simulink model "sim_discrete_planar_2dof"  (R2022b)
settings: Solver=VariableStepAuto  StartTime=0.0  StopTime=T_sim  FixedStep=param_global.Ta  RelTol=1e-3
InitFcn:
  | parameters_2dof;
undefined symbols (not defined in the file): coord_xaxis_len, coord_yaxis_len, coord_zaxis_len, fx, fy, fz, init_bus_param, param_global, param_path_set_points, param_robot, param_vis, path_coord_xaxis_len, path_coord_yaxis_len, path_coord_zaxis_len, path_param, q_0, q_0_p, tau_x, tau_y, tau_z, X, Y, Z
libraries: sm_lib x1241, nesl_utility x796
(part 1 of 34)
settings Model: Creator=emu  LastModifiedBy=emu
settings OptimizationCC: ReuseModelBlockBuffer=on
settings DebuggingCC: ReadBeforeWriteMsg=DisableAll  WriteAfterWriteMsg=DisableAll  WriteAfterReadMsg=DisableAll  MultiTaskDSMMsg=none
settings ModelReferenceCC: UpdateModelReferenceTargets=IfOutOfDate
settings SFSimCC: SFSimEcho=off

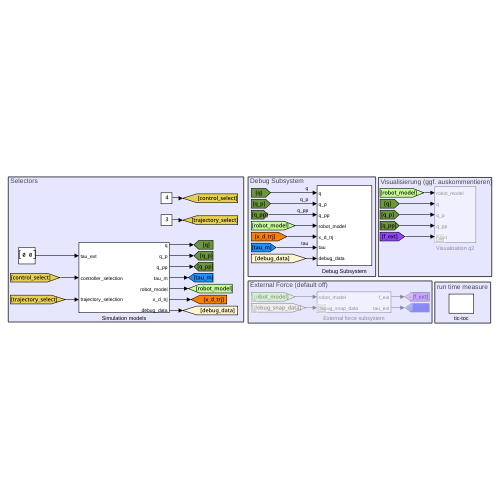
[thumbnail: Simulink's stored preview of the top-level diagram]
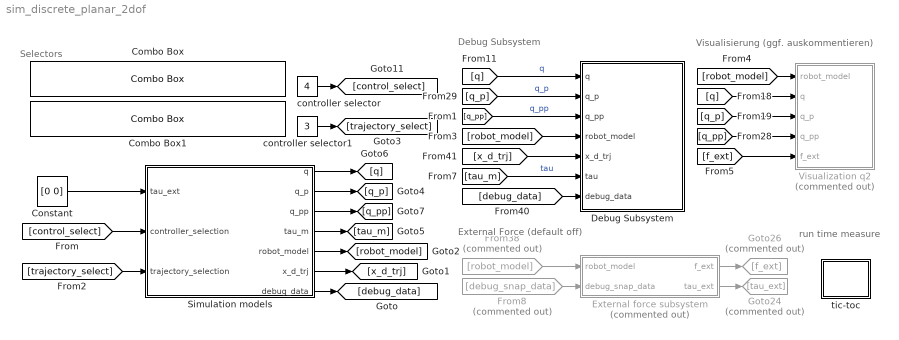
== system sim_discrete_planar_2dof ==
  note: run time measure
  note: Selectors
  note: Visualisierung (ggf. auskommentieren)
  note: Debug Subsystem
  note: External Force (default off)
  Combo Box : ComboBox  SelectedLabel=MPC 3 (qrqp, N=5, 50ms, v3)
      settings: Values={{'Cartesian CT Control', 'MPC 1 (ipopt, N=5, 20ms, v2)', 'MPC 2 (qrqp, N=5, 20ms, v2)', 'MPC 3 (qrqp, N=5, 50ms, v3)', 'MPC 4 (qrqp, N=5, 1ms, v1)', 'MPC 5 (qrqp, N=5, 10ms, v4'}, [1 2 3 4 5 6]}
  Combo Box1 : ComboBox  SelectedLabel=3: 5th order polynomial
      settings: Values={{'1: stabilize equilibrium', '2: 5th order differential filter', '3: 5th order polynomial', '4: smooth sinus'}, [1 2 3 4]}
  Constant : Constant  Value=[0 0]
  Debug Subsystem : Subsystem
  External force subsystem : Subsystem  Commented=on
  From38 : From  Commented=on  GotoTag=robot_model
  From8 : From  Commented=on  GotoTag=debug_snap_data
  Goto24 : Goto  Commented=on  GotoTag=tau_ext
  Goto26 : Goto  Commented=on  GotoTag=f_ext
  Simulation models : Subsystem
  Visualization q2 : Subsystem  Commented=on
  controller selector : Constant  Value=4  OutDataTypeStr=uint32  SampleTime=-1
  controller selector1 : Constant  Value=3  OutDataTypeStr=uint32  SampleTime=-1
  tic-toc : Subsystem
  wires:
  controller selector/1 -> [control_select]
  [tau_m] -> Debug Subsystem/6(tau)  "tau"
  [debug_data] -> Debug Subsystem/7(debug_data)
  External force subsystem/1(f_ext) -> Goto26/1
  [q_p] -> Debug Subsystem/2(q_p)  "q_p"
  [robot_model] -> Debug Subsystem/4(robot_model)
  [robot_model] -> Visualization q2/1(robot_model)
  From8/1 -> External force subsystem/2(debug_snap_data)
  [q_pp] -> Visualization q2/4(q_pp)
  [f_ext] -> Visualization q2/5(f_ext)
  [x_d_trj] -> Debug Subsystem/5(x_d_trj)
  From38/1 -> External force subsystem/1(robot_model)
  [q_p] -> Visualization q2/3(q_p)
  External force subsystem/2(tau_ext) -> Goto24/1
  [q] -> Visualization q2/2(q)
  [q] -> Debug Subsystem/1(q)  "q"
  [q_pp] -> Debug Subsystem/3(q_pp)  "q_pp"
  Simulation models/7(debug_data) -> [debug_data]
  Simulation models/6(x_d_trj) -> [x_d_trj]
  Simulation models/5(robot_model) -> [robot_model]
  Simulation models/4(tau_m) -> [tau_m]
  Simulation models/3(q_pp) -> [q_pp]
  Simulation models/2(q_p) -> [q_p]
  Simulation models/1(q) -> [q]
  [control_select] -> Simulation models/2(controller_selection)
  Constant/1 -> Simulation models/1(tau_ext)
  controller selector1/1 -> [trajectory_select]
  [trajectory_select] -> Simulation models/3(trajectory_selection)

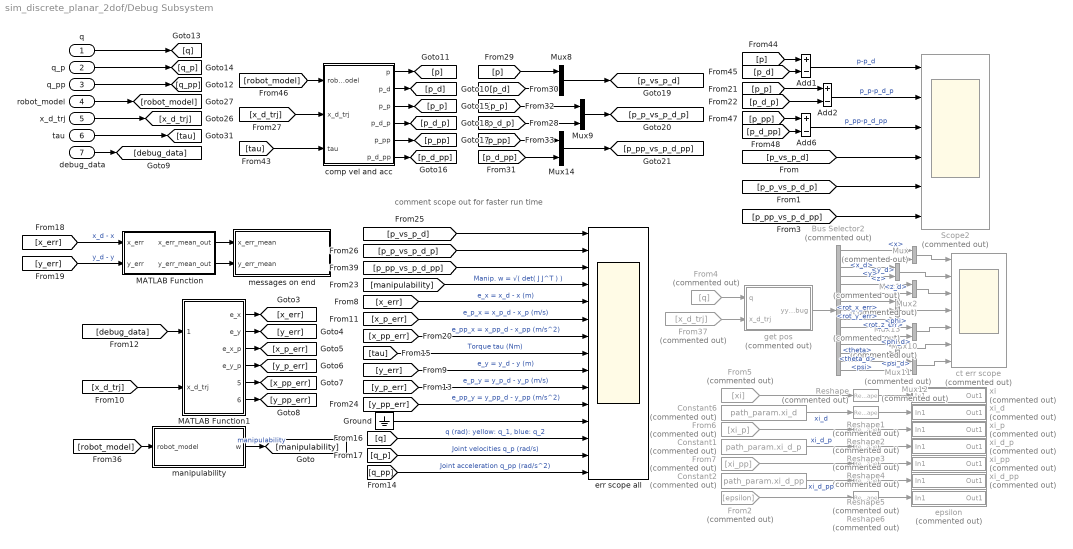
== system sim_discrete_planar_2dof/Debug Subsystem ==
  note: comment scope out for faster run time
  q : Inport
  q_p : Inport  Port=2
  q_pp : Inport  Port=3
  robot_model : Inport  Port=4
  x_d_trj : Inport  Port=5
  tau : Inport  Port=6
  debug_data : Inport  Port=7  OutDataTypeStr=Bus: debug_data_bus
  Add1 : Sum  Inputs=+-
  Add2 : Sum  Inputs=+-
  Add6 : Sum  Inputs=+-
  Bus Selector2 : BusSelector  Commented=on  OutputSignals=x,x_d,y,y_d,z,z_d,rot_x_err,rot_y_err,rot_z_err,phi,phi_d,theta,theta_d,psi,psi_d
  Constant1 : Constant  Commented=on  Value=path_param.xi_d_p  SampleTime=-1
  Constant2 : Constant  Commented=on  Value=path_param.xi_d_pp  SampleTime=-1
  Constant6 : Constant  Commented=on  Value=path_param.xi_d  SampleTime=-1
  From2 : From  Commented=on  GotoTag=epsilon
  From37 : From  Commented=on  GotoTag=x_d_trj
  From4 : From  Commented=on  GotoTag=q
  From5 : From  Commented=on  GotoTag=xi
  From6 : From  Commented=on  GotoTag=xi_p
  From7 : From  Commented=on  GotoTag=xi_pp
  Ground : Ground
  MATLAB Function : MATLAB Function  TreatAsAtomicUnit=on
  MATLAB Function1 : MATLAB Function  TreatAsAtomicUnit=on
  Mux{,1..2,10..12} (6 alike) : Mux  Commented=on  Inputs=2
  Mux13 : Mux  Commented=on  Inputs=3
  Mux14 : Mux  Inputs=2
  Mux8 : Mux  Inputs=2
  Mux9 : Mux  Inputs=2
  Reshape{,1..6} (7 alike) : Reshape  Commented=on
  Scope2 : Scope  Commented=on  NumInputPorts=6
  comp vel and acc : MATLAB Function  TreatAsAtomicUnit=on
  ct err scope : Scope  Commented=on  NumInputPorts=7
  epsilon : Subsystem  Commented=on
  err scope all : Scope  NumInputPorts=15
  get pos : MATLAB Function  Commented=on  TreatAsAtomicUnit=on  SystemSampleTime=param_global.Ta
  manipulability : MATLAB Function  TreatAsAtomicUnit=on
  messages on end : Subsystem
  xi : Subsystem  Commented=on
  xi_d : Subsystem  Commented=on
  xi_d_p : Subsystem  Commented=on
  xi_d_pp : Subsystem  Commented=on
  xi_p : Subsystem  Commented=on
  xi_pp : Subsystem  Commented=on
  wires:
  [robot_model] -> manipulability/1(robot_model)
  q/1 -> [q]
  q_p/1 -> [q_p]
  q_pp/1 -> [q_pp]
  From4/1 -> get pos/1(q)
  Constant6/1 -> Reshape1/1  "xi_d"
  Constant1/1 -> Reshape3/1  "xi_d_p"
  Constant2/1 -> Reshape5/1  "xi_d_pp"
  From5/1 -> Reshape/1
  From6/1 -> Reshape2/1
  From7/1 -> Reshape4/1
  x_d_trj/1 -> [x_d_trj]
  robot_model/1 -> [robot_model]
  debug_data/1 -> [debug_data]
  tau/1 -> [tau]
  From37/1 -> get pos/2(x_d_trj)
  get pos/1(yy_debug) -> Bus Selector2/1
  Mux/1 -> ct err scope/1
  Mux1/1 -> ct err scope/2
  Mux2/1 -> ct err scope/3
  Mux10/1 -> ct err scope/5
  Mux11/1 -> ct err scope/6
  Mux12/1 -> ct err scope/7
  Mux13/1 -> ct err scope/4
  Bus Selector2/1 -> Mux/1  "<x>"
  Bus Selector2/2 -> Mux/2  "<x_d>"
  Bus Selector2/3 -> Mux1/1  "<y>"
  Bus Selector2/4 -> Mux1/2  "<y_d>"
  Bus Selector2/5 -> Mux2/1  "<z>"
  Bus Selector2/6 -> Mux2/2  "<z_d>"
  Bus Selector2/7 -> Mux13/1  "<rot_x_err>"
  Bus Selector2/8 -> Mux13/2  "<rot_y_err>"
  Bus Selector2/9 -> Mux13/3  "<rot_z_err>"
  Bus Selector2/10 -> Mux10/1  "<phi>"
  Bus Selector2/11 -> Mux10/2  "<phi_d>"
  Bus Selector2/12 -> Mux11/1  "<theta>"
  Bus Selector2/13 -> Mux11/2  "<theta_d>"
  Bus Selector2/14 -> Mux12/1  "<psi>"
  Bus Selector2/15 -> Mux12/2  "<psi_d>"
  Reshape/1 -> xi/1(In1)
  Reshape1/1 -> xi_d/1(In1)
  Reshape2/1 -> xi_p/1(In1)
  Reshape3/1 -> xi_d_p/1(In1)
  Reshape4/1 -> xi_pp/1(In1)
  Reshape5/1 -> xi_d_pp/1(In1)
  From2/1 -> Reshape6/1
  Reshape6/1 -> epsilon/1(In1)
  manipulability/1(w) -> [manipulability]  "manipulability"
  [x_err] -> MATLAB Function/1(x_err)  "x_d - x"
  [y_err] -> MATLAB Function/2(y_err)  "y_d - y"
  MATLAB Function/2(y_err_mean_out) -> messages on end/2(y_err_mean)
  MATLAB Function/1(x_err_mean_out) -> messages on end/1(x_err_mean)
  [debug_data] -> MATLAB Function1/1(debug_data)
  [x_d_trj] -> MATLAB Function1/2(x_d_trj)
  MATLAB Function1/1(e_x) -> [x_err]
  MATLAB Function1/2(e_y) -> [y_err]
  MATLAB Function1/3(e_x_p) -> [x_p_err]
  MATLAB Function1/4(e_y_p) -> [y_p_err]
  MATLAB Function1/5(e_x_pp) -> [x_pp_err]
  MATLAB Function1/6(e_y_pp) -> [y_pp_err]
  [manipulability] -> err scope all/4  "Manip. w = √( det( J J^T ) )"
  [tau] -> err scope all/8  "Torque tau (Nm)"
  [x_err] -> err scope all/5  "e_x = x_d - x (m)"
  [y_err] -> err scope all/9  "e_y = y_d - y (m)"
  [x_p_err] -> err scope all/6  "e_p_x = x_p_d - x_p (m/s)"
  [y_p_err] -> err scope all/10  "e_p_y = y_p_d - y_p (m/s)"
  [x_pp_err] -> err scope all/7  "e_pp_x = x_pp_d - x_pp (m/s^2)"
  [y_pp_err] -> err scope all/11  "e_pp_y = y_pp_d - y_pp (m/s^2)"
  [q] -> err scope all/13  "q (rad): yellow: q_1, blue: q_2"
  [q_p] -> err scope all/14  "Joint velocities q_p (rad/s)"
  [q_pp] -> err scope all/15  "Joint acceleration q_pp (rad/s^2)"
  [p_d_p] -> Mux9/2
  [tau] -> comp vel and acc/3(tau)
  [p_p] -> Mux9/1
  [p_d] -> Mux8/2
  [x_d_trj] -> comp vel and acc/2(x_d_trj)
  [p] -> Mux8/1
  [p_pp] -> Mux14/1
  [p_d_pp] -> Mux14/2
  [robot_model] -> comp vel and acc/1(robot_model)
  comp vel and acc/2(p_d) -> [p_d]
  comp vel and acc/1(p) -> [p]
  comp vel and acc/3(p_p) -> [p_p]
  comp vel and acc/6(p_d_pp) -> [p_d_pp]
  comp vel and acc/5(p_pp) -> [p_pp]
  comp vel and acc/4(p_d_p) -> [p_d_p]
  [p_pp] -> Add6/1
  [p] -> Add1/1
  [p_d] -> Add1/2
  [p_d_p] -> Add2/2
  [p_p] -> Add2/1
  Add6/1 -> Scope2/3  "p_pp-p_d_pp"
  Add2/1 -> Scope2/2  "p_p-p_d_p"
  Add1/1 -> Scope2/1  "p-p_d"
  [p_d_pp] -> Add6/2
  [p_p_vs_p_d_p] -> err scope all/2
  [p_vs_p_d] -> err scope all/1
  [p_pp_vs_p_d_pp] -> err scope all/3
  Ground/1 -> err scope all/12
  Mux8/1 -> [p_vs_p_d]
  Mux9/1 -> [p_p_vs_p_d_p]
  Mux14/1 -> [p_pp_vs_p_d_pp]
  [p_vs_p_d] -> Scope2/4
  [p_p_vs_p_d_p] -> Scope2/5
  [p_pp_vs_p_d_pp] -> Scope2/6

== system sim_discrete_planar_2dof/External force subsystem ==
  note: Force basis system
  note: Manual force testing
  note: Auto force Function: used for video creation
  note: force multiplier
  note: Exogeneous Disturbance
  note: Pritzi force input
  robot_model : Inport
  debug_snap_data : Inport  Port=2  OutDataTypeStr=Bus: debug_snap_data_bus
  Con4 : Constant  Value=0  SampleTime=-1
  Constant : Constant  Value=0.1  SampleTime=-1
  Constant{1,5..8} (5 alike) : Constant  Value=0  SampleTime=-1
  Constant2 : Constant  Value=0.25  SampleTime=-1
  Constant4 : Constant  Value=0.5  SampleTime=-1
  Constant9 : Constant  SampleTime=-1
  Manual Switch{1..2,6,8} (4 alike) : ManualSwitch
  Manual Switch{3..5,9} (4 alike) : ManualSwitch  CurrentSetting=0
  Mux3 : Mux  Inputs=6
  NOT : Logic  Operator=NOT  IconShape=distinctive  AllPortsSameDT=off  OutDataTypeStr=boolean
  Product1 : Product
  Push Button1 : PushButtonBlock  ButtonText=fx  OnValue=20  OffValue=0.000000
  Push Button10 : PushButtonBlock  ButtonText=-tau_x  OnValue=-1  OffValue=0.000000
  Push Button11 : PushButtonBlock  ButtonText=-tau_y  OnValue=-1  OffValue=0.000000
  Push Button12 : PushButtonBlock  ButtonText=-tau_z  OnValue=-1  OffValue=0.000000
  Push Button2 : PushButtonBlock  ButtonText=fz  OnValue=20  OffValue=0.000000
  Push Button3 : PushButtonBlock  ButtonText=tau_z  OffValue=0.000000
  Push Button4 : PushButtonBlock  ButtonText=tau_y  OffValue=0.000000
  Push Button5 : PushButtonBlock  ButtonText=tau_x  OffValue=0.000000
  Push Button6 : PushButtonBlock  ButtonText=fy  OnValue=20  OffValue=0.000000
  Push Button7 : PushButtonBlock  ButtonText=-fx  OnValue=-20  OffValue=0.000000
  Push Button8 : PushButtonBlock  ButtonText=-fy  OnValue=-20  OffValue=0.000000
  Push Button9 : PushButtonBlock  ButtonText=-fz  OnValue=-20  OffValue=0.000000
  Switch : Switch  Criteria=u2 > Threshold  SaturateOnIntegerOverflow=off  InputSameDT=off
  Switch1 : Switch  Criteria=u2 > Threshold  SaturateOnIntegerOverflow=off  InputSameDT=off
  auto force : Constant  Value=0
  auto force functions : Subsystem
  external_force : MATLAB Function  TreatAsAtomicUnit=on
  f_e = f_E_E : Constant  Value=2  SampleTime=-1
  f_e = f_W_E : Constant  SampleTime=-1
  f_e = f_path : Constant  Value=3  SampleTime=-1
  manual force : Constant
  orientation snap test : Constant  Value=2
  path snap poly test : Constant
  path snap test : Constant  Value=3
  f_ext : Outport
  tau_ext : Outport  Port=2
  wires:
  Product1/1 -> [f_W_E]
  Constant4/1 -> Manual Switch3/1
  Constant6/1 -> Mux3/6
  Constant5/1 -> Mux3/3
  Constant/1 -> Manual Switch2/1
  Constant8/1 -> Mux3/4
  [f_W_E] -> external_force/1(f_W_E)
  external_force/2(f_ext) -> f_ext/1
  Constant9/1 -> Manual Switch3/2
  Manual Switch2/1 -> Manual Switch4/1
  Con4/1 -> Mux3/2
  [robot_model] -> external_force/2(robot_model)
  Manual Switch4/1 -> Product1/2
  Constant1/1 -> Mux3/1
  f_e = f_W_E/1 -> Manual Switch1/1
  Constant7/1 -> Mux3/5
  Constant2/1 -> Manual Switch2/2
  f_e = f_E_E/1 -> Manual Switch1/2
  Manual Switch3/1 -> Manual Switch4/2
  external_force/1(tau_ext) -> tau_ext/1
  Manual Switch1/1 -> Manual Switch5/1
  f_e = f_path/1 -> Manual Switch5/2
  robot_model/1 -> [robot_model]
  debug_snap_data/1 -> [debug_snap_data]
  [debug_snap_data] -> external_force/3(debug_snap_data)
  Switch1/1 -> Product1/1
  Manual Switch5/1 -> Switch/1
  [custom_mode] -> Switch/3
  auto force functions/1(y) -> [step_data]
  auto force functions/2(custom_mode) -> [custom_mode]
  Manual Switch6/1 -> auto force functions/1(snap selection)
  path snap test/1 -> Manual Switch8/2
  orientation snap test/1 -> Manual Switch8/1
  manual force/1 -> Manual Switch9/1
  auto force/1 -> Manual Switch9/2
  Manual Switch9/1 -> [force_mode]
  [force_mode] -> Switch/2
  [force_mode] -> Switch1/2
  [step_data] -> Switch1/3
  Mux3/1 -> Switch1/1
  Switch/1 -> [mode]
  [mode] -> external_force/4(mode)
  Manual Switch8/1 -> Manual Switch6/2
  path snap poly test/1 -> Manual Switch6/1
  [force_mode] -> NOT/1
  NOT/1 -> auto force functions/Enable

== system sim_discrete_planar_2dof/Simulation models ==
  note: Controller
  note: Trajectory (Umschaltzeit ist param_vis.T/2)
  note: Robot Model
  note: perfect measurement
  tau_ext : Inport
  controller_selection : Inport  Port=2
  trajectory_selection : Inport  Port=3
  Add : Sum
  Constant3 : Constant
  Constant6 : Constant  Value=0
  Create Sollfilter Trajektorie : Subsystem  ReferencedSubsystem=trajectory_generation_ref_subsys
  Robot model : Subsystem
  Robot model bus : MATLAB Function  TreatAsAtomicUnit=on
  controller : Subsystem
  q : Outport
  q_p : Outport  Port=2
  q_pp : Outport  Port=3
  tau_m : Outport  Port=4
  robot_model : Outport  Port=5  OutDataTypeStr=Bus: robot_model
  x_d_trj : Outport  Port=6  OutDataTypeStr=Bus: x_d
  debug_data : Outport  Port=7  OutDataTypeStr=Bus: debug_data_bus
  wires:
  [q_p] -> Robot model bus/2(q_p)
  Robot model/2(q_p) -> [q_p]
  [tau_ext] -> Add/2
  Robot model/3(q_pp) -> [q_pp]
  [q] -> Robot model bus/1(q)
  [q_pp] -> Robot model bus/3(q_pp)
  Robot model/1(q) -> [q]
  [robot_model] -> Create Sollfilter Trajektorie/1
  [tau] -> Add/1
  Robot model bus/1(robot_model) -> [robot_model]
  tau_ext/1 -> [tau_ext]
  controller_selection/1 -> [control_select]
  [q_pp] -> q_pp/1
  [q_p] -> q_p/1
  [x_d_trj_o] -> x_d_trj/1
  [debug_data] -> debug_data/1
  [q] -> q/1
  [robot_model] -> robot_model/1
  [tau] -> tau_m/1
  controller/3(debug_data) -> [debug_data]
  [robot_model] -> controller/1(robot_model)
  [control_select] -> controller/2(controller_selector)
  [x_d_trj_in] -> controller/4(x_d_trj)
  controller/1(tau) -> [tau]
  Constant3/1 -> Create Sollfilter Trajektorie/3
  Constant6/1 -> Create Sollfilter Trajektorie/5, Create Sollfilter Trajektorie/4
  Create Sollfilter Trajektorie/1 -> [x_d_trj_in]
  trajectory_selection/1 -> [trajectory_select]
  [trajectory_select] -> Create Sollfilter Trajektorie/2
  controller/2(x_d_trj_o) -> [x_d_trj_o]
  [trajectory_select] -> controller/3(trajectory selector)
  Add/1 -> Robot model/1(tau)

== system sim_discrete_planar_2dof/Visualization q2 ==
  robot_model : Inport  OutDataTypeStr=Bus: robot_model
  q : Inport  Port=2
  q_p : Inport  Port=3
  q_pp : Inport  Port=4
  f_ext : Inport  Port=5
  Constant : Constant  Commented=on
  Demux1 : Demux  Outputs=2
  Demux2 : Demux  Outputs=2
  Demux3 : Demux  Outputs=2
  KOS_World_xI : Subsystem
  KOS_endeffektor : Subsystem
  MechanismConfiguration1 : sm_lib/Utilities/Mechanism Configuration  UniformGravity=Constant  GravityVector=param_robot.g_vis  GravityVectorUnits=m/s^2  LinearizationDelta=0.001  JointModeTransitionNonlinearIterations=2
  Simulink-PS Converter : nesl_utility/Simulink-PS Converter  PhysicalDomain=network_engine_domain  SubClassName=ps_input  LeftPortType=input  RightPortType=output  PseudoPeriodic=off  Frequencies=[]  NoiseDistribution=none  NoiseParameters=[]  Unit=1  AffineConversion=off  FilteringAndDerivatives=provide  SimscapeFilterOrder=1  InputFilterTimeConstant=0.001  UdotUserProvided=2
  Simulink-PS Converter1 : nesl_utility/Simulink-PS Converter  PhysicalDomain=network_engine_domain  SubClassName=ps_input  LeftPortType=input  RightPortType=output  PseudoPeriodic=off  Frequencies=[]  NoiseDistribution=none  NoiseParameters=[]  Unit=1  AffineConversion=off  FilteringAndDerivatives=provide  SimscapeFilterOrder=1  InputFilterTimeConstant=0.001  UdotUserProvided=2
  Solver Configuration1 : nesl_utility/Solver Configuration  PhysicalDomain=network_engine_domain  SubClassName=solver  LeftPortType=input  RightPortType=generic  Tones=[]  Harmonics=[]  SimulateNoise=off  Profile=off  UseLocalSampling=off  EnableSwitchedLinearOptims=on  FrequencyDomain=off  UseCCode=off  RelTol=0.001  AbsTol=1e-06  MinStep=1e-09  ToWorkspace=off  RevertToSquareIcSolve=off  NumHomotopyIterations=100  PhasorMode=off  PhasorModeNumHarmonics=1  ConsistencySolver=NEWTON_FTOL  IndexReductionMethod=DerivativeReplacement  DoDC=off  ResidualTolerance=1e-09  ConsistencyTolSource=GLOBAL  ConsistencyAbsTol=0.001  ConsistencyRelTol=0.001  ConsistencyTolFactor=0.001  UseLocalSolver=off  LocalSolverChoice=NE_BACKWARD_EULER_ADVANCER  ResolveIndetEquations=on  FunctionEvalNumThread=1  LocalSolverSampleTime=0.001  DoFixedCost=off  MaxNonlinIter=3  MaxModeIter=2  ComputeImpulses=off  ImpulseIterations=2  LinearAlgebra=Sparse  LinearAlgebraNumThread=0  EquationFormulation=NE_TIME_EF  DelaysMemoryBudget=1024  AutomaticFiltering=on  FilteringTimeConstant=0.001  PartitionStorageMethod=AS_NEEDED  PartitionMemoryBudget=1024  PartitionMethod=FAST  MultibodyUseLocalSolver=off  MultibodyLocalSolverChoice=ODE1  MultibodyLocalSolverSampleTime=0.001
  Spur Endeffektor : Subsystem
  World : sm_lib/Frames and Transforms/World Frame
  base : Subsystem
  bulk 1 : Subsystem
  bulk 2 : Subsystem
  bulk1_offset : sm_lib/Frames and Transforms/Rigid Transform  TranslationMethod=Cartesian  TranslationStandardOffset=0  TranslationStandardOffsetUnits=m  TranslationStandardAxis=+Z  TranslationCartesianOffset=param_robot.link1_offset  TranslationCartesianOffsetUnits=m  TranslationROffset=0  TranslationROffsetUnits=m  TranslationZOffset=0  TranslationZOffsetUnits=m  TranslationThetaOffset=0  TranslationThetaOffsetUnits=deg  RotationMethod=RotationMatrix  RotationAngleUnits=deg  RotationStandardAxis=+Z  RotationAngle=0.0  RotationArbitraryAxis=[0 0 1]  FollAlignAxisA=+X  BaseAlignAxisA=+Y  FollAlignAxisB=+Y  BaseAlignAxisB=+Z  RotationSequenceAxes=BaseAxes  RotationSequence=XYZ  RotationSequenceAngles=[1.57079632679, 0, -3.141592653589587]  RotationSequenceAnglesUnits=rad  RotationMatrix=eye(3)  Quaternion=[1 0 0 0]
  bulk2_offset : sm_lib/Frames and Transforms/Rigid Transform  TranslationMethod=Cartesian  TranslationStandardOffset=0  TranslationStandardOffsetUnits=m  TranslationStandardAxis=+Z  TranslationCartesianOffset=param_robot.link2_offset  TranslationCartesianOffsetUnits=m  TranslationROffset=0  TranslationROffsetUnits=m  TranslationZOffset=0  TranslationZOffsetUnits=m  TranslationThetaOffset=0  TranslationThetaOffsetUnits=deg  RotationMethod=RotationMatrix  RotationAngleUnits=deg  RotationStandardAxis=+Z  RotationAngle=0.0  RotationArbitraryAxis=[0 0 1]  FollAlignAxisA=+X  BaseAlignAxisA=+Y  FollAlignAxisB=+Y  BaseAlignAxisB=+Z  RotationSequenceAxes=BaseAxes  RotationSequence=XYZ  RotationSequenceAngles=[1.57079632679, 0, -3.141592653589587]  RotationSequenceAnglesUnits=rad  RotationMatrix=eye(3)  Quaternion=[1 0 0 0]
  dynamic single path visualize line (slow) : Subsystem  Commented=on
  dynamic single path visualize spline (slow) : Subsystem  Commented=on
  joint 1 : sm_lib/Joints/Revolute Joint  JointMode=Normal  SenseConstraintForce=off  SenseConstraintForceX=off  SenseConstraintForceY=off  SenseConstraintForceZ=off  SenseConstraintTorque=off  SenseConstraintTorqueX=off  SenseConstraintTorqueY=off  SenseConstraintTorqueZ=off  SenseTotalForce=off  SenseTotalForceX=off  SenseTotalForceY=off  SenseTotalForceZ=off  SenseTotalTorque=off  SenseTotalTorqueX=off  SenseTotalTorqueY=off  SenseTotalTorqueZ=off  CompositeWrenchDir=FollowerOnBase  CompositeWrenchFrame=BaseFrame  EquilibriumPosition=0  EquilibriumPositionUnits=deg  SpringStiffness=0  SpringStiffnessUnits=N*m/deg  DampingCoefficient=0  DampingCoefficientUnits=m^2*kg/s/rad  TorqueActuationMode=ComputedTorque  MotionActuationMode=InputMotion  SensePosition=off  SenseVelocity=off  SenseAcceleration=off  SenseTorqueForce=off  SenseLowerLimitTorqueForce=off  SenseUpperLimitTorqueForce=off  LowerLimitSpecify=off  LowerLimitBound=-90  LowerLimitBoundUnits=deg  LowerLimitStiffness=1e4  LowerLimitStiffnessUnits=N*m/deg  LowerLimitDamping=10  LowerLimitDampingUnits=N*m/(deg/s)  LowerLimitTransitionRegionWidth=0.1  LowerLimitTransitionRegionWidthUnits=deg  UpperLimitSpecify=off  UpperLimitBound=90  UpperLimitBoundUnits=deg  UpperLimitStiffness=1e4  UpperLimitStiffnessUnits=N*m/deg  UpperLimitDamping=10  UpperLimitDampingUnits=N*m/(deg/s)  UpperLimitTransitionRegionWidth=0.1  UpperLimitTransitionRegionWidthUnits=deg  PositionTargetSpecify=on  PositionTargetPriority=High  PositionTargetValue=0  PositionTargetValueUnits=rad  VelocityTargetSpecify=off  VelocityTargetPriority=High  VelocityTargetValue=0  VelocityTargetValueUnits=deg/s
  joint 2 : sm_lib/Joints/Revolute Joint  JointMode=Normal  SenseConstraintForce=off  SenseConstraintForceX=off  SenseConstraintForceY=off  SenseConstraintForceZ=off  SenseConstraintTorque=off  SenseConstraintTorqueX=off  SenseConstraintTorqueY=off  SenseConstraintTorqueZ=off  SenseTotalForce=off  SenseTotalForceX=off  SenseTotalForceY=off  SenseTotalForceZ=off  SenseTotalTorque=off  SenseTotalTorqueX=off  SenseTotalTorqueY=off  SenseTotalTorqueZ=off  CompositeWrenchDir=FollowerOnBase  CompositeWrenchFrame=BaseFrame  EquilibriumPosition=0  EquilibriumPositionUnits=deg  SpringStiffness=0  SpringStiffnessUnits=N*m/deg  DampingCoefficient=0  DampingCoefficientUnits=m^2*kg/s/rad  TorqueActuationMode=ComputedTorque  MotionActuationMode=InputMotion  SensePosition=off  SenseVelocity=off  SenseAcceleration=off  SenseTorqueForce=off  SenseLowerLimitTorqueForce=off  SenseUpperLimitTorqueForce=off  LowerLimitSpecify=off  LowerLimitBound=-90  LowerLimitBoundUnits=deg  LowerLimitStiffness=1e4  LowerLimitStiffnessUnits=N*m/deg  LowerLimitDamping=10  LowerLimitDampingUnits=N*m/(deg/s)  LowerLimitTransitionRegionWidth=0.1  LowerLimitTransitionRegionWidthUnits=deg  UpperLimitSpecify=off  UpperLimitBound=90  UpperLimitBoundUnits=deg  UpperLimitStiffness=1e4  UpperLimitStiffnessUnits=N*m/deg  UpperLimitDamping=10  UpperLimitDampingUnits=N*m/(deg/s)  UpperLimitTransitionRegionWidth=0.1  UpperLimitTransitionRegionWidthUnits=deg  PositionTargetSpecify=on  PositionTargetPriority=High  PositionTargetValue=0  PositionTargetValueUnits=rad  VelocityTargetSpecify=off  VelocityTargetPriority=High  VelocityTargetValue=0  VelocityTargetValueUnits=deg/s
  joint_ee : sm_lib/Joints/Weld Joint  JointMode=Normal  SenseConstraintForce=off  SenseConstraintForceX=off  SenseConstraintForceY=off  SenseConstraintForceZ=off  SenseConstraintTorque=off  SenseConstraintTorqueX=off  SenseConstraintTorqueY=off  SenseConstraintTorqueZ=off  SenseTotalForce=off  SenseTotalForceX=off  SenseTotalForceY=off  SenseTotalForceZ=off  SenseTotalTorque=off  SenseTotalTorqueX=off  SenseTotalTorqueY=off  SenseTotalTorqueZ=off  CompositeWrenchDir=FollowerOnBase  CompositeWrenchFrame=BaseFrame
  show pritzi force arrows : Subsystem  Commented=on
  static single path visualize spline (fast) : Subsystem  Commented=on
  world : Subsystem
  world_base : sm_lib/Joints/Weld Joint  JointMode=Normal  SenseConstraintForce=off  SenseConstraintForceX=off  SenseConstraintForceY=off  SenseConstraintForceZ=off  SenseConstraintTorque=off  SenseConstraintTorqueX=off  SenseConstraintTorqueY=off  SenseConstraintTorqueZ=off  SenseTotalForce=off  SenseTotalForceX=off  SenseTotalForceY=off  SenseTotalForceZ=off  SenseTotalTorque=off  SenseTotalTorqueX=off  SenseTotalTorqueY=off  SenseTotalTorqueZ=off  CompositeWrenchDir=FollowerOnBase  CompositeWrenchFrame=BaseFrame
  wires:
  q/1 -> [q], Demux3/1
  [q1] -> Simulink-PS Converter1/1
  robot_model/1 -> Spur Endeffektor/1(robot_model)
  q_p/1 -> Demux1/1
  q_pp/1 -> Demux2/1
  [q1p] -> Simulink-PS Converter1/2
  [q1pp] -> Simulink-PS Converter1/3
  [q2pp] -> Simulink-PS Converter/3
  [q2p] -> Simulink-PS Converter/2
  [q2] -> Simulink-PS Converter/1
  f_ext/1 -> show pritzi force arrows/2(f_ext)
  Constant/1 -> dynamic single path visualize spline (slow)/1(path_set)
  Demux2/1 -> [q1pp]
  Demux2/2 -> [q2pp]
  Demux3/1 -> [q1]
  Demux3/2 -> [q2]
  Demux1/1 -> [q1p]
  Demux1/2 -> [q2p]
  [q] -> show pritzi force arrows/1(q)
  connections:
  world/LConn1 -- World/RConn1 -- Solver Configuration1/RConn1 -- MechanismConfiguration1/RConn1
  joint 2/LConn1 -- bulk 1/RConn1
  bulk1_offset/RConn1 -- bulk 1/LConn1
  world/RConn1 -- static single path visualize spline (fast)/LConn1 -- Spur Endeffektor/LConn1 -- world_base/LConn1
  bulk2_offset/RConn1 -- bulk 2/LConn1
  base/RConn1 -- joint 1/LConn1
  Simulink-PS Converter1/RConn1 -- joint 1/LConn2
  Simulink-PS Converter/RConn1 -- joint 2/LConn2
  KOS_World_xI/LConn1 -- world_base/RConn1 -- base/LConn1
  KOS_endeffektor/LConn1 -- joint_ee/RConn1
  bulk 2/RConn1 -- joint_ee/LConn1
  joint 1/RConn1 -- bulk1_offset/LConn1
  joint 2/RConn1 -- bulk2_offset/LConn1

== system sim_discrete_planar_2dof/tic-toc ==
  note: tic-toc v1
  note: tic-toc v2
  Add : Sum  Inputs=+-
  Add1 : Sum  Inputs=+-
  Add2 : Sum
  Clock : Clock  Commented=on
  MATLAB Function : MATLAB Function  Commented=on  TreatAsAtomicUnit=on
  Step : Step  Time=param_global.Ta*2  SampleTime=param_global.Ta
  Step1 : Step  Time=param_global.Ta  SampleTime=param_global.Ta
  Step2 : Step  Time=param_vis.T-param_global.Ta  SampleTime=param_global.Ta
  Step3 : Step  Time=param_vis.T  SampleTime=param_global.Ta
  tic-toc : Subsystem  TreatAsAtomicUnit=on
  wires:
  Add/1 -> Add2/2  "start"
  Step2/1 -> Add1/1
  Add2/1 -> tic-toc/Trigger
  Step3/1 -> Add1/2
  Add1/1 -> Add2/1  "end"
  Step1/1 -> Add/1
  Step/1 -> Add/2
  Clock/1 -> MATLAB Function/1(t)

== system sim_discrete_planar_2dof/Debug Subsystem/epsilon ==
  In1 : Inport
  Out1 : Outport
  wires:
  In1/1 -> Out1/1

== system sim_discrete_planar_2dof/Debug Subsystem/messages on end ==
  note: tic-toc v1
  note: output debug text on end
  x_err_mean : Inport
  y_err_mean : Inport  Port=2
  Add1 : Sum  Inputs=+-
  Clock : Clock  Commented=on
  MATLAB Function : MATLAB Function  Commented=on  TreatAsAtomicUnit=on
  Step2 : Step  Time=param_vis.T-param_global.Ta  SampleTime=param_global.Ta
  Step3 : Step  Time=param_vis.T  SampleTime=param_global.Ta
  output debug text : Subsystem  TreatAsAtomicUnit=on
  wires:
  Clock/1 -> MATLAB Function/1(t)
  Add1/1 -> output debug text/Trigger  "end"
  Step3/1 -> Add1/2
  Step2/1 -> Add1/1
  y_err_mean/1 -> output debug text/2(y_err_mean)
  x_err_mean/1 -> output debug text/1(x_err_mean)

== system sim_discrete_planar_2dof/Debug Subsystem/xi ==
  In1 : Inport
  Out1 : Outport
  wires:
  In1/1 -> Out1/1

== system sim_discrete_planar_2dof/Debug Subsystem/xi_d ==
  In1 : Inport
  Out1 : Outport
  wires:
  In1/1 -> Out1/1

== system sim_discrete_planar_2dof/Debug Subsystem/xi_d_p ==
  In1 : Inport
  Out1 : Outport
  wires:
  In1/1 -> Out1/1

== system sim_discrete_planar_2dof/Debug Subsystem/xi_d_pp ==
  In1 : Inport
  Out1 : Outport
  wires:
  In1/1 -> Out1/1

== system sim_discrete_planar_2dof/Debug Subsystem/xi_p ==
  In1 : Inport
  Out1 : Outport
  wires:
  In1/1 -> Out1/1

== system sim_discrete_planar_2dof/Debug Subsystem/xi_pp ==
  In1 : Inport
  Out1 : Outport
  wires:
  In1/1 -> Out1/1

== system sim_discrete_planar_2dof/External force subsystem/auto force functions ==
  note: Orientation snap tests, (path_param.pathset == 4, Welder Aufsatz)
  note: Path snap tests (path_param.pathset == 4, Welder Aufsatz)
  note: Settings 3 Polynom Paths without endeffector
      | (path_param.pathset == 1, kein Aufsatz)
  snap selection : Inport
  Enable : EnablePort
  Clock : Clock
  Clock1 : Clock
  Clock2 : Clock
  Constant24 : Constant  Value=40
  Constant25 : Constant  Value=[10 0 0 0 0 0]
  Constant26 : Constant  Value=42
  Constant27 : Constant  Value=[15 0 0 0 0 0]
  Constant28 : Constant  Value=41
  Constant29 : Constant  Value=75
  Constant30 : Constant  Value=[-10 0 0 0 0 0]
  Constant31 : Constant  Value=78
  Constant32 : Constant  Value=[-15 0 0 0 0 0]
  Constant33 : Constant  Value=77
  Constant34 : Constant  Value=43
  Constant35 : Constant  Value=115
  Constant36 : Constant  Value=[10 0 0 0 0 0]
  Constant37 : Constant  Value=80
  Constant38 : Constant  Value=0
  If1 : If  IfExpression=u1 == 1  ElseIfExpressions=u1 == 2
  Merge3 : Merge  Inputs=3
  Merge4 : Merge  Inputs=3
  forces : Constant  Value=|
      | [0 2 0 0 0 0;
      | 0 0 2 0 0 0;
      | 0 -2 0 0 0 0;
      | 0 0 -2 0 0 0;
      | 30 0 7 0 0 0;
      | 0 2 0 0 0 0;
      | 0 0 2 0 0 0;
      | 0 -2 0 0 0 0;
      | 0 0 -2 0 0 0;
      | 0 0 0 0 0 0;
      | 0 0 0 0.25 0 0;
      | 0 0 0 0.26 0 0;
      | 0 0 0 0.27 0 0;
      | 0 0 0 0 0 0;
      | 0 -2 0 0 0 0;
      | 0 0 0 0 0 0;
      | 0 0 0 0.26 0 0;
      | 0 0 0 0 0 0;
      | 0 -2 0 0 0 0;
      | 0 0 2 0 0 0;
      | 0 2 0 0 0 0;
      | 0 0 -2 0 0 0;
      | -30 3.5 -3.5 0 0 0;
      | 0 -2 0 0 0 0;
      | 0 0 2 0 0 0;
      | 0 2 0 0 0 0;
      | 0 0 -2 0 0 0;
      | 0 0 0 0 0 0;
      | 0 0 0 0 0 0;
      | 0 0 0 0 0 0;
      | 0 0 0 0 0 0;
      | 0 0 0 0 0 0;
      | 0 0 0 0 0 0;
      | 0 0 0 0 0 0]
  forces1 : Constant  Value=|
      | [4.8 0 0 0 0 0;
      | 0 0 0 0 0 0;
      | 10 0 0 0 0 0;
      | 15 0 3 0 0 0
      | 10 0 3 0 0 0;
      | 0 0 0 0 0 0;
      | 1 0 0 0 0 0;
      | 0 0 0 0 0 0;
      | 15 0 0 0 0 0;
      | 10 -3 0 0 0 0
      | 10 0 0 0 0 0;
      | 0 0 0 0 0 0;
      | 1 0 0 0 0 0;
      | 0 0 0 0 0 0]
  get signals : MATLAB Function  TreatAsAtomicUnit=on
  get signals1 : MATLAB Function  TreatAsAtomicUnit=on
  get signals2 : MATLAB Function  TreatAsAtomicUnit=on
  m5 : Constant  Value=3
  m6 : Constant
  m7 : Constant  Value=3
  m8 : Constant
  m9 : Constant  Value=3
  modes : Constant  Value=[1 1 1 1 1 1 1 1 1 1 1 1 1 1 1 1 1 1 1 1 1 1 1 1 1 1 1 1 1 1 1 1 1 1]
  modes1 : Constant  Value=[3 3 1 1 1 3 3 1 1 1 1 3 3 3]
  t_start : Constant  Value="[0 1 2 3 4  5 6 7 8 9 10 10.3 10.5 10.7 11 12 13 13.5 15 16 17 18 19 20 21 22 23 24 25 26 27 28 29 30]"
  t_start1 : Constant  Value="[0 0.5 1.5 2 2.3  2.7 3.3 4 5.5  5.8 6.4 6.5 9 14.8]"
  use path poly : Subsystem  TreatAsAtomicUnit=on
  use welder path ori : Subsystem  TreatAsAtomicUnit=on
  use welder path snap : Subsystem  TreatAsAtomicUnit=on
  y : Outport
  custom_mode : Outport  Port=2
  wires:
  Constant26/1 -> get signals1/7(t1_e)
  Constant36/1 -> get signals1/20(y4)
  Constant34/1 -> get signals1/10(t2_a)
  Constant29/1 -> get signals1/11(t2_e)
  Constant37/1 -> get signals1/18(t4_a)
  Constant33/1 -> get signals1/14(t3_a)
  Constant35/1 -> get signals1/19(t4_e)
  Clock/1 -> get signals/1(t)
  m5/1 -> get signals1/5(m0)
  m8/1 -> get signals1/17(m3)
  m7/1 -> get signals1/13(m2)
  m9/1 -> get signals1/21(m4)
  Constant32/1 -> get signals1/16(y3)
  Clock1/1 -> get signals1/1(t)
  m6/1 -> get signals1/9(m1)
  Constant25/1 -> get signals1/4(y0)
  Constant38/1 -> get signals1/2(t0_a)
  Constant31/1 -> get signals1/15(t3_e)
  Constant28/1 -> get signals1/6(t1_a)
  Constant27/1 -> get signals1/8(y1)
  Constant24/1 -> get signals1/3(t0_e)
  Constant30/1 -> get signals1/12(y2)
  use path poly/1(Out1) -> Merge3/1
  use welder path ori/1(Out1) -> Merge3/2
  use path poly/2(Out2) -> Merge4/1
  use welder path ori/2(Out2) -> Merge4/2
  Merge4/1 -> custom_mode/1
  Merge3/1 -> y/1
  If1/1 -> use path poly/Action
  t_start/1 -> get signals/2(t0)
  forces/1 -> get signals/3(y0)
  modes/1 -> get signals/4(m0)
  t_start1/1 -> get signals2/2(t0)
  forces1/1 -> get signals2/3(y0)
  modes1/1 -> get signals2/4(m0)
  Clock2/1 -> get signals2/1(t)
  snap selection/1 -> If1/1
  If1/2 -> use welder path ori/Action
  If1/3 -> use welder path snap/Action
  use welder path snap/1(Out1) -> Merge3/3
  use welder path snap/2(Out2) -> Merge4/3
  get signals1/1(y) -> [y_poly]
  get signals1/2(custom_mode) -> [mode_poly]
  get signals2/1(y) -> [y_snap]
  get signals2/2(custom_mode) -> [mode_snap]
  get signals/1(y) -> [y_ori]
  get signals/2(custom_mode) -> [mode_ori]
  [y_poly] -> use path poly/1(y)
  [mode_poly] -> use path poly/2(In2)
  [y_ori] -> use welder path ori/1(custom_mode)
  [mode_ori] -> use welder path ori/2(In2)
  [y_snap] -> use welder path snap/1(custom_mode)
  [mode_snap] -> use welder path snap/2(In2)

== system sim_discrete_planar_2dof/Simulation models/Robot model ==
  tau : Inport
  Integrator : Integrator  InitialCondition=q_0
  Integrator1 : Integrator  InitialCondition=q_0_p
  Memory : Memory  InitialCondition=zeros(2,1)  InheritSampleTime=on
  Reduziertes System : MATLAB Function
  q : Outport
  q_p : Outport  Port=2
  q_pp : Outport  Port=3
  wires:
  Integrator/1 -> q/1, Reduziertes System/2(q)
  Integrator1/1 -> q_p/1, Reduziertes System/3(q_p), Integrator/1  "q_p"
  Reduziertes System/1(q_pp) -> Memory/1, Integrator1/1  "q_pp"
  tau/1 -> Reduziertes System/1(tau)
  Memory/1 -> q_pp/1

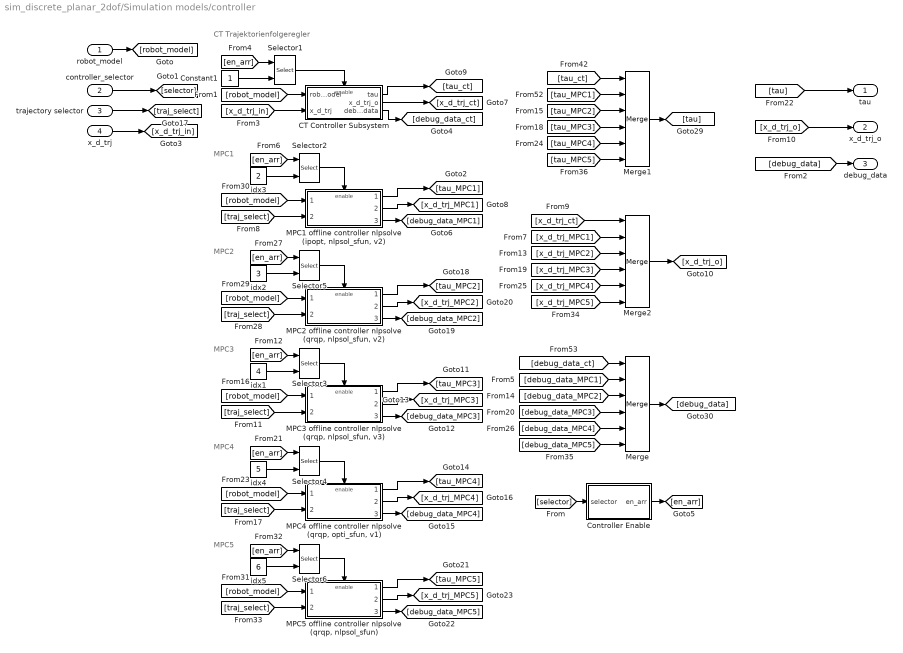
== system sim_discrete_planar_2dof/Simulation models/controller ==
  note: CT Trajektorienfolgeregler
  note: MPC1
  note: MPC2
  note: MPC3
  note: MPC4
  note: MPC5
  robot_model : Inport  OutDataTypeStr=Bus: robot_model
  controller_selector : Inport  Port=2
  trajectory selector : Inport  Port=3
  x_d_trj : Inport  Port=4  OutDataTypeStr=Bus: x_d
  CT Controller Subsystem : Subsystem
  Constant1 : Constant
  Controller Enable : MATLAB Function  TreatAsAtomicUnit=on
  "MPC1 offline controller nlpsolve (ipopt, nlpsol_sfun, v2)" : Subsystem  ReferencedSubsystem=MPC1_nlpsol
  "MPC2 offline controller nlpsolve (qrqp, nlpsol_sfun, v2)" : Subsystem  ReferencedSubsystem=MPC2_opti
  "MPC3 offline controller nlpsolve (qrqp, nlpsol_sfun, v3)" : Subsystem  ReferencedSubsystem=MPC3_opti
  "MPC4 offline controller nlpsolve (qrqp, opti_sfun, v1)" : Subsystem  ReferencedSubsystem=MPC4_opti
  "MPC5 offline controller nlpsolve (qrqp, nlpsol_sfun)" : Subsystem  ReferencedSubsystem=MPC5_nlpsol
  Merge : Merge  Inputs=6
  Merge1 : Merge  Inputs=6
  Merge2 : Merge  Inputs=6
  Selector{1..6} (6 alike) : Selector  IndexOptions=Index vector (port)  Indices=[1 3]  OutputSizes=1
  idx1 : Constant  Value=4
  idx2 : Constant  Value=3
  idx3 : Constant  Value=2
  idx4 : Constant  Value=5
  idx5 : Constant  Value=6
  tau : Outport
  x_d_trj_o : Outport  Port=2  OutDataTypeStr=Bus: x_d
  debug_data : Outport  Port=3  OutDataTypeStr=Bus: debug_data_bus
  wires:
  [robot_model] -> CT Controller Subsystem/1(robot_model)
  [debug_data] -> debug_data/1
  controller_selector/1 -> [selector]
  [tau] -> tau/1
  x_d_trj/1 -> [x_d_trj_in]
  [x_d_trj_in] -> CT Controller Subsystem/2(x_d_trj)
  Controller Enable/1(en_arr) -> [en_arr]
  [selector] -> Controller Enable/1(selector)
  robot_model/1 -> [robot_model]
  [debug_data_ct] -> Merge/1
  [tau_ct] -> Merge1/1
  [tau_MPC1] -> Merge1/2
  Merge1/1 -> [tau]
  Merge/1 -> [debug_data]
  [debug_data_MPC1] -> Merge/2
  CT Controller Subsystem/1(tau) -> [tau_ct]
  Selector1/1 -> CT Controller Subsystem/Enable
  Constant1/1 -> Selector1/2
  [en_arr] -> Selector1/1
  CT Controller Subsystem/3(debug_data) -> [debug_data_ct]
  CT Controller Subsystem/2(x_d_trj_o) -> [x_d_trj_ct]
  [x_d_trj_ct] -> Merge2/1
  [x_d_trj_MPC1] -> Merge2/2
  Merge2/1 -> [x_d_trj_o]
  [x_d_trj_o] -> x_d_trj_o/1
  [tau_MPC2] -> Merge1/3
  [debug_data_MPC2] -> Merge/3
  [x_d_trj_MPC2] -> Merge2/3
  [debug_data_MPC3] -> Merge/4
  [x_d_trj_MPC3] -> Merge2/4
  [tau_MPC3] -> Merge1/4
  [tau_MPC4] -> Merge1/5
  [x_d_trj_MPC4] -> Merge2/5
  [debug_data_MPC4] -> Merge/5
  trajectory selector/1 -> [traj_select]
  [traj_select] -> "MPC1 offline controller nlpsolve (ipopt, nlpsol_sfun, v2)"/2
  [en_arr] -> Selector2/1
  Selector2/1 -> "MPC1 offline controller nlpsolve (ipopt, nlpsol_sfun, v2)"/Enable
  "MPC1 offline controller nlpsolve (ipopt, nlpsol_sfun, v2)"/1 -> [tau_MPC1]
  "MPC1 offline controller nlpsolve (ipopt, nlpsol_sfun, v2)"/2 -> [x_d_trj_MPC1]
  idx3/1 -> Selector2/2
  "MPC1 offline controller nlpsolve (ipopt, nlpsol_sfun, v2)"/3 -> [debug_data_MPC1]
  [robot_model] -> "MPC1 offline controller nlpsolve (ipopt, nlpsol_sfun, v2)"/1
  idx2/1 -> Selector5/2
  [robot_model] -> "MPC2 offline controller nlpsolve (qrqp, nlpsol_sfun, v2)"/1
  [en_arr] -> Selector5/1
  Selector5/1 -> "MPC2 offline controller nlpsolve (qrqp, nlpsol_sfun, v2)"/Enable
  "MPC2 offline controller nlpsolve (qrqp, nlpsol_sfun, v2)"/2 -> [x_d_trj_MPC2]
  "MPC2 offline controller nlpsolve (qrqp, nlpsol_sfun, v2)"/1 -> [tau_MPC2]
  [traj_select] -> "MPC2 offline controller nlpsolve (qrqp, nlpsol_sfun, v2)"/2
  "MPC2 offline controller nlpsolve (qrqp, nlpsol_sfun, v2)"/3 -> [debug_data_MPC2]
  [robot_model] -> "MPC3 offline controller nlpsolve (qrqp, nlpsol_sfun, v3)"/1
  [en_arr] -> Selector3/1
  "MPC3 offline controller nlpsolve (qrqp, nlpsol_sfun, v3)"/2 -> [x_d_trj_MPC3]
  [traj_select] -> "MPC3 offline controller nlpsolve (qrqp, nlpsol_sfun, v3)"/2
  "MPC3 offline controller nlpsolve (qrqp, nlpsol_sfun, v3)"/3 -> [debug_data_MPC3]
  "MPC3 offline controller nlpsolve (qrqp, nlpsol_sfun, v3)"/1 -> [tau_MPC3]
  Selector3/1 -> "MPC3 offline controller nlpsolve (qrqp, nlpsol_sfun, v3)"/Enable
  idx1/1 -> Selector3/2
  [en_arr] -> Selector4/1
  "MPC4 offline controller nlpsolve (qrqp, opti_sfun, v1)"/2 -> [x_d_trj_MPC4]
  Selector4/1 -> "MPC4 offline controller nlpsolve (qrqp, opti_sfun, v1)"/Enable
  "MPC4 offline controller nlpsolve (qrqp, opti_sfun, v1)"/1 -> [tau_MPC4]
  "MPC4 offline controller nlpsolve (qrqp, opti_sfun, v1)"/3 -> [debug_data_MPC4]
  idx4/1 -> Selector4/2
  [robot_model] -> "MPC4 offline controller nlpsolve (qrqp, opti_sfun, v1)"/1
  [traj_select] -> "MPC4 offline controller nlpsolve (qrqp, opti_sfun, v1)"/2
  idx5/1 -> Selector6/2
  "MPC5 offline controller nlpsolve (qrqp, nlpsol_sfun)"/1 -> [tau_MPC5]
  Selector6/1 -> "MPC5 offline controller nlpsolve (qrqp, nlpsol_sfun)"/Enable
  "MPC5 offline controller nlpsolve (qrqp, nlpsol_sfun)"/3 -> [debug_data_MPC5]
  [traj_select] -> "MPC5 offline controller nlpsolve (qrqp, nlpsol_sfun)"/2
  "MPC5 offline controller nlpsolve (qrqp, nlpsol_sfun)"/2 -> [x_d_trj_MPC5]
  [en_arr] -> Selector6/1
  [robot_model] -> "MPC5 offline controller nlpsolve (qrqp, nlpsol_sfun)"/1
  [debug_data_MPC5] -> Merge/6
  [x_d_trj_MPC5] -> Merge2/6
  [tau_MPC5] -> Merge1/6

== system sim_discrete_planar_2dof/Visualization q2/KOS_World_xI ==
  x_I : sm_lib/Curves and Surfaces/Spline  GraphicAmbientColor=[0.15 0.15 0.15 1.0]  GraphicDiffuseColor=coord_xaxis_color  DataPoints=[0 coord_xaxis_len; 0 0; 0 0]'  DataPointsUnits=cm  GraphicEmissiveColor=[0.0 0.0 0.0 1.0]  EndConditionType=Natural  MarkerShape=Sphere  MarkerSize=10  GraphicType=FromGeometry  GraphicOpacity=coord_xaxis_opacity  GraphicShininess=75  GraphicSpecularColor=[0.5 0.5 0.5 1.0]  GraphicVisPropType=SimpleVisualProperties
  y_I : sm_lib/Curves and Surfaces/Spline  GraphicAmbientColor=[0.15 0.15 0.15 1.0]  GraphicDiffuseColor=coord_yaxis_color  DataPoints=[0 0; 0 coord_yaxis_len; 0 0]'  DataPointsUnits=cm  GraphicEmissiveColor=[0.0 0.0 0.0 1.0]  EndConditionType=Natural  MarkerShape=Sphere  MarkerSize=10  GraphicType=FromGeometry  GraphicOpacity=coord_yaxis_opacity  GraphicShininess=75  GraphicSpecularColor=[0.5 0.5 0.5 1.0]  GraphicVisPropType=SimpleVisualProperties
  z_I : sm_lib/Curves and Surfaces/Spline  GraphicAmbientColor=[0.15 0.15 0.15 1.0]  GraphicDiffuseColor=coord_zaxis_color  DataPoints=[0 0; 0 0; 0 coord_zaxis_len]'  DataPointsUnits=cm  GraphicEmissiveColor=[0.0 0.0 0.0 1.0]  EndConditionType=Natural  MarkerShape=Sphere  MarkerSize=10  GraphicType=FromGeometry  GraphicOpacity=coord_zaxis_opacity  GraphicShininess=75  GraphicSpecularColor=[0.5 0.5 0.5 1.0]  GraphicVisPropType=SimpleVisualProperties
  Conn1 : PMIOPort  Side=Left
  connections:
  z_I/LConn1 -- y_I/LConn1 -- x_I/LConn1 -- Conn1/RConn1

== system sim_discrete_planar_2dof/Visualization q2/KOS_endeffektor ==
  x_e : sm_lib/Curves and Surfaces/Spline  GraphicAmbientColor=[0.15 0.15 0.15 1.0]  GraphicDiffuseColor=coord_xaxis_color  DataPoints=[0 coord_xaxis_len; 0 0; 0 0]'  DataPointsUnits=cm  GraphicEmissiveColor=[0.0 0.0 0.0 1.0]  EndConditionType=Natural  MarkerShape=Sphere  MarkerSize=10  GraphicType=FromGeometry  GraphicOpacity=coord_xaxis_opacity  GraphicShininess=75  GraphicSpecularColor=[0.5 0.5 0.5 1.0]  GraphicVisPropType=SimpleVisualProperties
  y_e : sm_lib/Curves and Surfaces/Spline  GraphicAmbientColor=[0.15 0.15 0.15 1.0]  GraphicDiffuseColor=coord_yaxis_color  DataPoints=[0 0; 0 coord_yaxis_len; 0 0]'  DataPointsUnits=cm  GraphicEmissiveColor=[0.0 0.0 0.0 1.0]  EndConditionType=Natural  MarkerShape=Sphere  MarkerSize=10  GraphicType=FromGeometry  GraphicOpacity=coord_yaxis_opacity  GraphicShininess=75  GraphicSpecularColor=[0.5 0.5 0.5 1.0]  GraphicVisPropType=SimpleVisualProperties
  z_e : sm_lib/Curves and Surfaces/Spline  GraphicAmbientColor=[0.15 0.15 0.15 1.0]  GraphicDiffuseColor=coord_zaxis_color  DataPoints=[0 0; 0 0; 0 coord_zaxis_len]'  DataPointsUnits=cm  GraphicEmissiveColor=[0.0 0.0 0.0 1.0]  EndConditionType=Natural  MarkerShape=Sphere  MarkerSize=10  GraphicType=FromGeometry  GraphicOpacity=coord_zaxis_opacity  GraphicShininess=75  GraphicSpecularColor=[0.5 0.5 0.5 1.0]  GraphicVisPropType=SimpleVisualProperties
  Conn1 : PMIOPort  Side=Left
  connections:
  Conn1/RConn1 -- x_e/LConn1 -- z_e/LConn1 -- y_e/LConn1

== system sim_discrete_planar_2dof/Visualization q2/Spur Endeffektor ==
  robot_model : Inport
  Add : Sum  Commented=on
  Add1 : Sum  Commented=on
  From5 : From  Commented=on  GotoTag=start1
  From6 : From  Commented=on  GotoTag=delta
  From7 : From  Commented=on  GotoTag=start2
  From8 : From  Commented=on  GotoTag=delta
  Goto4 : Goto  Commented=on  GotoTag=start2
  Goto5 : Goto  Commented=on  GotoTag=start3
  MATLAB Function : MATLAB Function  TreatAsAtomicUnit=on
  Product : Product  Commented=on
  Product1 : Product  Commented=on
  Visualisierung Punkte : Subsystem
  const : Constant  Commented=on  Value=100
  const1 : Constant  Commented=on  Value=100
  delta : Constant  Value=param_vis.vis_point.delta
  start : Constant  SampleTime=-1
  Conn1 : PMIOPort  Side=Left
  wires:
  MATLAB Function/1(p1) -> Visualisierung Punkte/1(p1)
  MATLAB Function/2(p2) -> Visualisierung Punkte/2(p2)
  MATLAB Function/3(p3) -> Visualisierung Punkte/3(p3)
  MATLAB Function/4(p4) -> Visualisierung Punkte/4(p4)
  MATLAB Function/5(p5) -> Visualisierung Punkte/5(p5)
  MATLAB Function/6(p6) -> Visualisierung Punkte/6(p6)
  MATLAB Function/7(p7) -> Visualisierung Punkte/7(p7)
  MATLAB Function/8(p8) -> Visualisierung Punkte/8(p8)
  MATLAB Function/9(p9) -> Visualisierung Punkte/9(p9)
  MATLAB Function/10(p10) -> Visualisierung Punkte/10(p10)
  MATLAB Function/11(p11) -> Visualisierung Punkte/11(p11)
  MATLAB Function/12(p12) -> Visualisierung Punkte/12(p12)
  MATLAB Function/13(p13) -> Visualisierung Punkte/13(p13)
  MATLAB Function/14(p14) -> Visualisierung Punkte/14(p14)
  MATLAB Function/15(p15) -> Visualisierung Punkte/15(p15)
  MATLAB Function/16(p16) -> Visualisierung Punkte/16(p16)
  MATLAB Function/17(p17) -> Visualisierung Punkte/17(p17)
  MATLAB Function/18(p18) -> Visualisierung Punkte/18(p18)
  MATLAB Function/19(p19) -> Visualisierung Punkte/19(p19)
  MATLAB Function/20(p20) -> Visualisierung Punkte/20(p20)
  MATLAB Function/21(p21) -> Visualisierung Punkte/21(p21)
  MATLAB Function/22(p22) -> Visualisierung Punkte/22(p22)
  MATLAB Function/23(p23) -> Visualisierung Punkte/23(p23)
  MATLAB Function/24(p24) -> Visualisierung Punkte/24(p24)
  MATLAB Function/25(p25) -> Visualisierung Punkte/25(p25)
  MATLAB Function/26(p26) -> Visualisierung Punkte/26(p26)
  MATLAB Function/27(p27) -> Visualisierung Punkte/27(p27)
  MATLAB Function/28(p28) -> Visualisierung Punkte/28(p28)
  MATLAB Function/29(p29) -> Visualisierung Punkte/29(p29)
  MATLAB Function/30(p30) -> Visualisierung Punkte/30(p30)
  MATLAB Function/31(p31) -> Visualisierung Punkte/31(p31)
  MATLAB Function/32(p32) -> Visualisierung Punkte/32(p32)
  MATLAB Function/33(p33) -> Visualisierung Punkte/33(p33)
  MATLAB Function/34(p34) -> Visualisierung Punkte/34(p34)
  MATLAB Function/35(p35) -> Visualisierung Punkte/35(p35)
  MATLAB Function/36(p36) -> Visualisierung Punkte/36(p36)
  MATLAB Function/37(p37) -> Visualisierung Punkte/37(p37)
  MATLAB Function/38(p38) -> Visualisierung Punkte/38(p38)
  MATLAB Function/39(p39) -> Visualisierung Punkte/39(p39)
  MATLAB Function/40(p40) -> Visualisierung Punkte/40(p40)
  MATLAB Function/41(p41) -> Visualisierung Punkte/41(p41)
  MATLAB Function/42(p42) -> Visualisierung Punkte/42(p42)
  MATLAB Function/43(p43) -> Visualisierung Punkte/43(p43)
  MATLAB Function/44(p44) -> Visualisierung Punkte/44(p44)
  MATLAB Function/45(p45) -> Visualisierung Punkte/45(p45)
  MATLAB Function/46(p46) -> Visualisierung Punkte/46(p46)
  MATLAB Function/47(p47) -> Visualisierung Punkte/47(p47)
  MATLAB Function/48(p48) -> Visualisierung Punkte/48(p48)
  MATLAB Function/49(p49) -> Visualisierung Punkte/49(p49)
  MATLAB Function/50(p50) -> Visualisierung Punkte/50(p50)
  MATLAB Function/51(p51) -> Visualisierung Punkte/51(p51)
  MATLAB Function/52(p52) -> Visualisierung Punkte/52(p52)
  MATLAB Function/53(p53) -> Visualisierung Punkte/53(p53)
  MATLAB Function/54(p54) -> Visualisierung Punkte/54(p54)
  MATLAB Function/55(p55) -> Visualisierung Punkte/55(p55)
  MATLAB Function/56(p56) -> Visualisierung Punkte/56(p56)
  MATLAB Function/57(p57) -> Visualisierung Punkte/57(p57)
  MATLAB Function/58(p58) -> Visualisierung Punkte/58(p58)
  MATLAB Function/59(p59) -> Visualisierung Punkte/59(p59)
  MATLAB Function/60(p60) -> Visualisierung Punkte/60(p60)
  MATLAB Function/61(p61) -> Visualisierung Punkte/61(p61)
  MATLAB Function/62(p62) -> Visualisierung Punkte/62(p62)
  MATLAB Function/63(p63) -> Visualisierung Punkte/63(p63)
  MATLAB Function/64(p64) -> Visualisierung Punkte/64(p64)
  MATLAB Function/65(p65) -> Visualisierung Punkte/65(p65)
  MATLAB Function/66(p66) -> Visualisierung Punkte/66(p66)
  MATLAB Function/67(p67) -> Visualisierung Punkte/67(p67)
  MATLAB Function/68(p68) -> Visualisierung Punkte/68(p68)
  MATLAB Function/69(p69) -> Visualisierung Punkte/69(p69)
  MATLAB Function/70(p70) -> Visualisierung Punkte/70(p70)
  MATLAB Function/71(p71) -> Visualisierung Punkte/71(p71)
  MATLAB Function/72(p72) -> Visualisierung Punkte/72(p72)
  MATLAB Function/73(p73) -> Visualisierung Punkte/73(p73)
  MATLAB Function/74(p74) -> Visualisierung Punkte/74(p74)
  MATLAB Function/75(p75) -> Visualisierung Punkte/75(p75)
  MATLAB Function/76(p76) -> Visualisierung Punkte/76(p76)
  MATLAB Function/77(p77) -> Visualisierung Punkte/77(p77)
  MATLAB Function/78(p78) -> Visualisierung Punkte/78(p78)
  MATLAB Function/79(p79) -> Visualisierung Punkte/79(p79)
  MATLAB Function/80(p80) -> Visualisierung Punkte/80(p80)
  MATLAB Function/81(p81) -> Visualisierung Punkte/81(p81)
  MATLAB Function/82(p82) -> Visualisierung Punkte/82(p82)
  MATLAB Function/83(p83) -> Visualisierung Punkte/83(p83)
  MATLAB Function/84(p84) -> Visualisierung Punkte/84(p84)
  MATLAB Function/85(p85) -> Visualisierung Punkte/85(p85)
  MATLAB Function/86(p86) -> Visualisierung Punkte/86(p86)
  MATLAB Function/87(p87) -> Visualisierung Punkte/87(p87)
  MATLAB Function/88(p88) -> Visualisierung Punkte/88(p88)
  MATLAB Function/89(p89) -> Visualisierung Punkte/89(p89)
  MATLAB Function/90(p90) -> Visualisierung Punkte/90(p90)
  MATLAB Function/91(p91) -> Visualisierung Punkte/91(p91)
  MATLAB Function/92(p92) -> Visualisierung Punkte/92(p92)
  MATLAB Function/93(p93) -> Visualisierung Punkte/93(p93)
  MATLAB Function/94(p94) -> Visualisierung Punkte/94(p94)
  MATLAB Function/95(p95) -> Visualisierung Punkte/95(p95)
  MATLAB Function/96(p96) -> Visualisierung Punkte/96(p96)
  MATLAB Function/97(p97) -> Visualisierung Punkte/97(p97)
  MATLAB Function/98(p98) -> Visualisierung Punkte/98(p98)
  MATLAB Function/99(p99) -> Visualisierung Punkte/99(p99)
  MATLAB Function/100(p100) -> Visualisierung Punkte/100(p100)
  [delta] -> MATLAB Function/3(delta)
  robot_model/1 -> [robot_model]
  [robot_model] -> MATLAB Function/1(robot_model)
  delta/1 -> [delta]
  [start1] -> MATLAB Function/2(start)
  start/1 -> [start1]
  From5/1 -> Add/1
  Add/1 -> Goto4/1
  Product/1 -> Add/2
  const/1 -> Product/1
  From6/1 -> Product/2
  From8/1 -> Product1/2
  const1/1 -> Product1/1
  Product1/1 -> Add1/2
  From7/1 -> Add1/1
  Add1/1 -> Goto5/1
  connections:
  Visualisierung Punkte/LConn1 -- Conn1/RConn1
